simulink model "rfidet_2048ch_600mhzv2"  (R2013b) (continued)
== system rfidet_2048ch_600mhzv2 == (continued)
      | port_label('output', 1, 'q');
      | disp(['Z^-', num2str(latency)]);
  pipeline57 : Subsystem  AncestorBlock=casper_library_delays/pipeline  mask(pipeline){latency=1}
      mask help:
      | An explicitly laid-out delay line to avoid mapping to SRL16s
      | for use in pipelining to help achieve timing closure.
      mask init:
      | pipeline_init(gcb,'latency',latency);
      settings: AttributesFormatString=Latency = %<latency>
      mask Display:
      | port_label('input', 1, 'd');
      | port_label('output', 1, 'q');
      | disp(['Z^-', num2str(latency)]);
  pipeline58 : Subsystem  AncestorBlock=casper_library_delays/pipeline  mask(pipeline){latency=1}
      mask help:
      | An explicitly laid-out delay line to avoid mapping to SRL16s
      | for use in pipelining to help achieve timing closure.
      mask init:
      | pipeline_init(gcb,'latency',latency);
      settings: AttributesFormatString=Latency = %<latency>
      mask Display:
      | port_label('input', 1, 'd');
      | port_label('output', 1, 'q');
      | disp(['Z^-', num2str(latency)]);
  pipeline59 : Subsystem  AncestorBlock=casper_library_delays/pipeline  mask(pipeline){latency=1}
      mask help:
      | An explicitly laid-out delay line to avoid mapping to SRL16s
      | for use in pipelining to help achieve timing closure.
      mask init:
      | pipeline_init(gcb,'latency',latency);
      settings: AttributesFormatString=Latency = %<latency>
      mask Display:
      | port_label('input', 1, 'd');
      | port_label('output', 1, 'q');
      | disp(['Z^-', num2str(latency)]);
  pipeline6 : Subsystem  AncestorBlock=casper_library_delays/pipeline  mask(pipeline){latency=2}
      mask help:
      | An explicitly laid-out delay line to avoid mapping to SRL16s
      | for use in pipelining to help achieve timing closure.
      mask init:
      | pipeline_init(gcb,'latency',latency);
      settings: AttributesFormatString=Latency = %<latency>
      mask Display:
      | port_label('input', 1, 'd');
      | port_label('output', 1, 'q');
      | disp(['Z^-', num2str(latency)]);
  pipeline60 : Subsystem  AncestorBlock=casper_library_delays/pipeline  mask(pipeline){latency=1}
      mask help:
      | An explicitly laid-out delay line to avoid mapping to SRL16s
      | for use in pipelining to help achieve timing closure.
      mask init:
      | pipeline_init(gcb,'latency',latency);
      settings: AttributesFormatString=Latency = %<latency>
      mask Display:
      | port_label('input', 1, 'd');
      | port_label('output', 1, 'q');
      | disp(['Z^-', num2str(latency)]);
  pipeline61 : Subsystem  AncestorBlock=casper_library_delays/pipeline  mask(pipeline){latency=2}
      mask help:
      | An explicitly laid-out delay line to avoid mapping to SRL16s
      | for use in pipelining to help achieve timing closure.
      mask init:
      | pipeline_init(gcb,'latency',latency);
      settings: AttributesFormatString=Latency = %<latency>
      mask Display:
      | port_label('input', 1, 'd');
      | port_label('output', 1, 'q');
      | disp(['Z^-', num2str(latency)]);
  pipeline62 : Subsystem  AncestorBlock=casper_library_delays/pipeline  mask(pipeline){latency=2}
      mask help:
      | An explicitly laid-out delay line to avoid mapping to SRL16s
      | for use in pipelining to help achieve timing closure.
      mask init:
      | pipeline_init(gcb,'latency',latency);
      settings: AttributesFormatString=Latency = %<latency>
      mask Display:
      | port_label('input', 1, 'd');
      | port_label('output', 1, 'q');
      | disp(['Z^-', num2str(latency)]);
  pipeline63 : Subsystem  AncestorBlock=casper_library_delays/pipeline  mask(pipeline){latency=1}
      mask help:
      | An explicitly laid-out delay line to avoid mapping to SRL16s
      | for use in pipelining to help achieve timing closure.
      mask init:
      | pipeline_init(gcb,'latency',latency);
      settings: AttributesFormatString=Latency = %<latency>
      mask Display:
      | port_label('input', 1, 'd');
      | port_label('output', 1, 'q');
      | disp(['Z^-', num2str(latency)]);
  pipeline64 : Subsystem  AncestorBlock=casper_library_delays/pipeline  mask(pipeline){latency=1}
      mask help:
      | An explicitly laid-out delay line to avoid mapping to SRL16s
      | for use in pipelining to help achieve timing closure.
      mask init:
      | pipeline_init(gcb,'latency',latency);
      settings: AttributesFormatString=Latency = %<latency>
      mask Display:
      | port_label('input', 1, 'd');
      | port_label('output', 1, 'q');
      | disp(['Z^-', num2str(latency)]);
  pipeline65 : Subsystem  AncestorBlock=casper_library_delays/pipeline  mask(pipeline){latency=2}
      mask help:
      | An explicitly laid-out delay line to avoid mapping to SRL16s
      | for use in pipelining to help achieve timing closure.
      mask init:
      | pipeline_init(gcb,'latency',latency);
      settings: AttributesFormatString=Latency = %<latency>
      mask Display:
      | port_label('input', 1, 'd');
      | port_label('output', 1, 'q');
      | disp(['Z^-', num2str(latency)]);
  pipeline66 : Subsystem  AncestorBlock=casper_library_delays/pipeline  mask(pipeline){latency=2}
      mask help:
      | An explicitly laid-out delay line to avoid mapping to SRL16s
      | for use in pipelining to help achieve timing closure.
      mask init:
      | pipeline_init(gcb,'latency',latency);
      settings: AttributesFormatString=Latency = %<latency>
      mask Display:
      | port_label('input', 1, 'd');
      | port_label('output', 1, 'q');
      | disp(['Z^-', num2str(latency)]);
  pipeline67 : Subsystem  AncestorBlock=casper_library_delays/pipeline  mask(pipeline){latency=2}
      mask help:
      | An explicitly laid-out delay line to avoid mapping to SRL16s
      | for use in pipelining to help achieve timing closure.
      mask init:
      | pipeline_init(gcb,'latency',latency);
      settings: AttributesFormatString=Latency = %<latency>
      mask Display:
      | port_label('input', 1, 'd');
      | port_label('output', 1, 'q');
      | disp(['Z^-', num2str(latency)]);
  pipeline68 : Subsystem  AncestorBlock=casper_library_delays/pipeline  mask(pipeline){latency=2}
      mask help:
      | An explicitly laid-out delay line to avoid mapping to SRL16s
      | for use in pipelining to help achieve timing closure.
      mask init:
      | pipeline_init(gcb,'latency',latency);
      settings: AttributesFormatString=Latency = %<latency>
      mask Display:
      | port_label('input', 1, 'd');
      | port_label('output', 1, 'q');
      | disp(['Z^-', num2str(latency)]);
  pipeline69 : Subsystem  AncestorBlock=casper_library_delays/pipeline  mask(pipeline){latency=1}
      mask help:
      | An explicitly laid-out delay line to avoid mapping to SRL16s
      | for use in pipelining to help achieve timing closure.
      mask init:
      | pipeline_init(gcb,'latency',latency);
      settings: AttributesFormatString=Latency = %<latency>
      mask Display:
      | port_label('input', 1, 'd');
      | port_label('output', 1, 'q');
      | disp(['Z^-', num2str(latency)]);
  pipeline7 : Subsystem  AncestorBlock=casper_library_delays/pipeline  mask(pipeline){latency=1}
      mask help:
      | An explicitly laid-out delay line to avoid mapping to SRL16s
      | for use in pipelining to help achieve timing closure.
      mask init:
      | pipeline_init(gcb,'latency',latency);
      settings: AttributesFormatString=Latency = %<latency>
      mask Display:
      | port_label('input', 1, 'd');
      | port_label('output', 1, 'q');
      | disp(['Z^-', num2str(latency)]);
  pipeline70 : Subsystem  AncestorBlock=casper_library_delays/pipeline  mask(pipeline){latency=2}
      mask help:
      | An explicitly laid-out delay line to avoid mapping to SRL16s
      | for use in pipelining to help achieve timing closure.
      mask init:
      | pipeline_init(gcb,'latency',latency);
      settings: AttributesFormatString=Latency = %<latency>
      mask Display:
      | port_label('input', 1, 'd');
      | port_label('output', 1, 'q');
      | disp(['Z^-', num2str(latency)]);
  pipeline71 : Subsystem  AncestorBlock=casper_library_delays/pipeline  mask(pipeline){latency=2}
      mask help:
      | An explicitly laid-out delay line to avoid mapping to SRL16s
      | for use in pipelining to help achieve timing closure.
      mask init:
      | pipeline_init(gcb,'latency',latency);
      settings: AttributesFormatString=Latency = %<latency>
      mask Display:
      | port_label('input', 1, 'd');
      | port_label('output', 1, 'q');
      | disp(['Z^-', num2str(latency)]);
  pipeline72 : Subsystem  AncestorBlock=casper_library_delays/pipeline  mask(pipeline){latency=1}
      mask help:
      | An explicitly laid-out delay line to avoid mapping to SRL16s
      | for use in pipelining to help achieve timing closure.
      mask init:
      | pipeline_init(gcb,'latency',latency);
      settings: AttributesFormatString=Latency = %<latency>
      mask Display:
      | port_label('input', 1, 'd');
      | port_label('output', 1, 'q');
      | disp(['Z^-', num2str(latency)]);
  pipeline73 : Subsystem  AncestorBlock=casper_library_delays/pipeline  mask(pipeline){latency=1}
      mask help:
      | An explicitly laid-out delay line to avoid mapping to SRL16s
      | for use in pipelining to help achieve timing closure.
      mask init:
      | pipeline_init(gcb,'latency',latency);
      settings: AttributesFormatString=Latency = %<latency>
      mask Display:
      | port_label('input', 1, 'd');
      | port_label('output', 1, 'q');
      | disp(['Z^-', num2str(latency)]);
  pipeline77 : Subsystem  AncestorBlock=casper_library_delays/pipeline  mask(pipeline){latency=2}
      mask help:
      | An explicitly laid-out delay line to avoid mapping to SRL16s
      | for use in pipelining to help achieve timing closure.
      mask init:
      | pipeline_init(gcb,'latency',latency);
      settings: AttributesFormatString=Latency = %<latency>
      mask Display:
      | port_label('input', 1, 'd');
      | port_label('output', 1, 'q');
      | disp(['Z^-', num2str(latency)]);
  pipeline79 : Subsystem  AncestorBlock=casper_library_delays/pipeline  mask(pipeline){latency=1}
      mask help:
      | An explicitly laid-out delay line to avoid mapping to SRL16s
      | for use in pipelining to help achieve timing closure.
      mask init:
      | pipeline_init(gcb,'latency',latency);
      settings: AttributesFormatString=Latency = %<latency>
      mask Display:
      | port_label('input', 1, 'd');
      | port_label('output', 1, 'q');
      | disp(['Z^-', num2str(latency)]);
  pipeline8 : Subsystem  AncestorBlock=casper_library_delays/pipeline  mask(pipeline){latency=1}
      mask help:
      | An explicitly laid-out delay line to avoid mapping to SRL16s
      | for use in pipelining to help achieve timing closure.
      mask init:
      | pipeline_init(gcb,'latency',latency);
      settings: AttributesFormatString=Latency = %<latency>
      mask Display:
      | port_label('input', 1, 'd');
      | port_label('output', 1, 'q');
      | disp(['Z^-', num2str(latency)]);
  pipeline80 : Subsystem  AncestorBlock=casper_library_delays/pipeline  mask(pipeline){latency=1}
      mask help:
      | An explicitly laid-out delay line to avoid mapping to SRL16s
      | for use in pipelining to help achieve timing closure.
      mask init:
      | pipeline_init(gcb,'latency',latency);
      settings: AttributesFormatString=Latency = %<latency>
      mask Display:
      | port_label('input', 1, 'd');
      | port_label('output', 1, 'q');
      | disp(['Z^-', num2str(latency)]);
  pipeline81 : Subsystem  AncestorBlock=casper_library_delays/pipeline  mask(pipeline){latency=1}
      mask help:
      | An explicitly laid-out delay line to avoid mapping to SRL16s
      | for use in pipelining to help achieve timing closure.
      mask init:
      | pipeline_init(gcb,'latency',latency);
      settings: AttributesFormatString=Latency = %<latency>
      mask Display:
      | port_label('input', 1, 'd');
      | port_label('output', 1, 'q');
      | disp(['Z^-', num2str(latency)]);
  pipeline82 : Subsystem  AncestorBlock=casper_library_delays/pipeline  mask(pipeline){latency=2}
      mask help:
      | An explicitly laid-out delay line to avoid mapping to SRL16s
      | for use in pipelining to help achieve timing closure.
      mask init:
      | pipeline_init(gcb,'latency',latency);
      settings: AttributesFormatString=Latency = %<latency>
      mask Display:
      | port_label('input', 1, 'd');
      | port_label('output', 1, 'q');
      | disp(['Z^-', num2str(latency)]);
  pipeline83 : Subsystem  AncestorBlock=casper_library_delays/pipeline  mask(pipeline){latency=1}
      mask help:
      | An explicitly laid-out delay line to avoid mapping to SRL16s
      | for use in pipelining to help achieve timing closure.
      mask init:
      | pipeline_init(gcb,'latency',latency);
      settings: AttributesFormatString=Latency = %<latency>
      mask Display:
      | port_label('input', 1, 'd');
      | port_label('output', 1, 'q');
      | disp(['Z^-', num2str(latency)]);
  pipeline84 : Subsystem  AncestorBlock=casper_library_delays/pipeline  mask(pipeline){latency=1}
      mask help:
      | An explicitly laid-out delay line to avoid mapping to SRL16s
      | for use in pipelining to help achieve timing closure.
      mask init:
      | pipeline_init(gcb,'latency',latency);
      settings: AttributesFormatString=Latency = %<latency>
      mask Display:
      | port_label('input', 1, 'd');
      | port_label('output', 1, 'q');
      | disp(['Z^-', num2str(latency)]);
  pipeline85 : Subsystem  AncestorBlock=casper_library_delays/pipeline  mask(pipeline){latency=2}
      mask help:
      | An explicitly laid-out delay line to avoid mapping to SRL16s
      | for use in pipelining to help achieve timing closure.
      mask init:
      | pipeline_init(gcb,'latency',latency);
      settings: AttributesFormatString=Latency = %<latency>
      mask Display:
      | port_label('input', 1, 'd');
      | port_label('output', 1, 'q');
      | disp(['Z^-', num2str(latency)]);
  pipeline87 : Subsystem  AncestorBlock=casper_library_delays/pipeline  mask(pipeline){latency=1}
      mask help:
      | An explicitly laid-out delay line to avoid mapping to SRL16s
      | for use in pipelining to help achieve timing closure.
      mask init:
      | pipeline_init(gcb,'latency',latency);
      settings: AttributesFormatString=Latency = %<latency>
      mask Display:
      | port_label('input', 1, 'd');
      | port_label('output', 1, 'q');
      | disp(['Z^-', num2str(latency)]);
  pipeline88 : Subsystem  AncestorBlock=casper_library_delays/pipeline  mask(pipeline){latency=1}
      mask help:
      | An explicitly laid-out delay line to avoid mapping to SRL16s
      | for use in pipelining to help achieve timing closure.
      mask init:
      | pipeline_init(gcb,'latency',latency);
      settings: AttributesFormatString=Latency = %<latency>
      mask Display:
      | port_label('input', 1, 'd');
      | port_label('output', 1, 'q');
      | disp(['Z^-', num2str(latency)]);
  pipeline89 : Subsystem  AncestorBlock=casper_library_delays/pipeline  mask(pipeline){latency=1}
      mask help:
      | An explicitly laid-out delay line to avoid mapping to SRL16s
      | for use in pipelining to help achieve timing closure.
      mask init:
      | pipeline_init(gcb,'latency',latency);
      settings: AttributesFormatString=Latency = %<latency>
      mask Display:
      | port_label('input', 1, 'd');
      | port_label('output', 1, 'q');
      | disp(['Z^-', num2str(latency)]);
  pipeline9 : Subsystem  AncestorBlock=casper_library_delays/pipeline  mask(pipeline){latency=1}
      mask help:
      | An explicitly laid-out delay line to avoid mapping to SRL16s
      | for use in pipelining to help achieve timing closure.
      mask init:
      | pipeline_init(gcb,'latency',latency);
      settings: AttributesFormatString=Latency = %<latency>
      mask Display:
      | port_label('input', 1, 'd');
      | port_label('output', 1, 'q');
      | disp(['Z^-', num2str(latency)]);
  pipeline90 : Subsystem  AncestorBlock=casper_library_delays/pipeline  mask(pipeline){latency=1}
      mask help:
      | An explicitly laid-out delay line to avoid mapping to SRL16s
      | for use in pipelining to help achieve timing closure.
      mask init:
      | pipeline_init(gcb,'latency',latency);
      settings: AttributesFormatString=Latency = %<latency>
      mask Display:
      | port_label('input', 1, 'd');
      | port_label('output', 1, 'q');
      | disp(['Z^-', num2str(latency)]);
  pipeline91 : Subsystem  AncestorBlock=casper_library_delays/pipeline  mask(pipeline){latency=2}
      mask help:
      | An explicitly laid-out delay line to avoid mapping to SRL16s
      | for use in pipelining to help achieve timing closure.
      mask init:
      | pipeline_init(gcb,'latency',latency);
      settings: AttributesFormatString=Latency = %<latency>
      mask Display:
      | port_label('input', 1, 'd');
      | port_label('output', 1, 'q');
      | disp(['Z^-', num2str(latency)]);
  pipeline92 : Subsystem  AncestorBlock=casper_library_delays/pipeline  mask(pipeline){latency=1}
      mask help:
      | An explicitly laid-out delay line to avoid mapping to SRL16s
      | for use in pipelining to help achieve timing closure.
      mask init:
      | pipeline_init(gcb,'latency',latency);
      settings: AttributesFormatString=Latency = %<latency>
      mask Display:
      | port_label('input', 1, 'd');
      | port_label('output', 1, 'q');
      | disp(['Z^-', num2str(latency)]);
  pipeline93 : Subsystem  AncestorBlock=casper_library_delays/pipeline  mask(pipeline){latency=1}
      mask help:
      | An explicitly laid-out delay line to avoid mapping to SRL16s
      | for use in pipelining to help achieve timing closure.
      mask init:
      | pipeline_init(gcb,'latency',latency);
      settings: AttributesFormatString=Latency = %<latency>
      mask Display:
      | port_label('input', 1, 'd');
      | port_label('output', 1, 'q');
      | disp(['Z^-', num2str(latency)]);
  pipeline94 : Subsystem  AncestorBlock=casper_library_delays/pipeline  mask(pipeline){latency=2}
      mask help:
      | An explicitly laid-out delay line to avoid mapping to SRL16s
      | for use in pipelining to help achieve timing closure.
      mask init:
      | pipeline_init(gcb,'latency',latency);
      settings: AttributesFormatString=Latency = %<latency>
      mask Display:
      | port_label('input', 1, 'd');
      | port_label('output', 1, 'q');
      | disp(['Z^-', num2str(latency)]);
  pipeline96 : Subsystem  AncestorBlock=casper_library_delays/pipeline  mask(pipeline){latency=1}
      mask help:
      | An explicitly laid-out delay line to avoid mapping to SRL16s
      | for use in pipelining to help achieve timing closure.
      mask init:
      | pipeline_init(gcb,'latency',latency);
      settings: AttributesFormatString=Latency = %<latency>
      mask Display:
      | port_label('input', 1, 'd');
      | port_label('output', 1, 'q');
      | disp(['Z^-', num2str(latency)]);
  pipeline97 : Subsystem  AncestorBlock=casper_library_delays/pipeline  mask(pipeline){latency=1}
      mask help:
      | An explicitly laid-out delay line to avoid mapping to SRL16s
      | for use in pipelining to help achieve timing closure.
      mask init:
      | pipeline_init(gcb,'latency',latency);
      settings: AttributesFormatString=Latency = %<latency>
      mask Display:
      | port_label('input', 1, 'd');
      | port_label('output', 1, 'q');
      | disp(['Z^-', num2str(latency)]);
  pipeline98 : Subsystem  AncestorBlock=casper_library_delays/pipeline  mask(pipeline){latency=2}
      mask help:
      | An explicitly laid-out delay line to avoid mapping to SRL16s
      | for use in pipelining to help achieve timing closure.
      mask init:
      | pipeline_init(gcb,'latency',latency);
      settings: AttributesFormatString=Latency = %<latency>
      mask Display:
      | port_label('input', 1, 'd');
      | port_label('output', 1, 'q');
      | disp(['Z^-', num2str(latency)]);
  pipeline99 : Subsystem  AncestorBlock=casper_library_delays/pipeline  mask(pipeline){latency=1}
      mask help:
      | An explicitly laid-out delay line to avoid mapping to SRL16s
      | for use in pipelining to help achieve timing closure.
      mask init:
      | pipeline_init(gcb,'latency',latency);
      settings: AttributesFormatString=Latency = %<latency>
      mask Display:
      | port_label('input', 1, 'd');
      | port_label('output', 1, 'q');
      | disp(['Z^-', num2str(latency)]);
  power{1..7,12} (8 alike) : casper_library_misc/power  BitWidth=18  add_latency=2  mult_latency=3
  signed_shifter{,1..15} (16 alike) : xbsIndex_r4/Black Box  init_code=shifter_signed_config  sim_method=Inactive  verbose=off  block_type=blackbox2
  simple_bram_vacc0_0 : Subsystem  AncestorBlock=casper_library_accumulators/simple_bram_vacc  mask(simple_bram_vacc){vec_len=512, arith_type=Unsigned, n_bits=64, bin_pt=35}
      mask prompts: vec_len "Vector length:"; arith_type "Output type:"; n_bits "Bit width (output):"; bin_pt "Binary point (output):"
      mask help: This simple vector accumulator is based on a shift register. It outputs its previous accumulated vector after a new_acc pulse is received.
      mask init:
      | simple_bram_vacc_init(gcb, ...
      |     'vec_len', vec_len, ...
      |     'arith_type', arith_type, ...
      |     'n_bits', n_bits, ...
      |     'bin_pt', bin_pt);
      mask TypeOptions arith_type: Signed | Unsigned
  simple_bram_vacc0_1 : Subsystem  AncestorBlock=casper_library_accumulators/simple_bram_vacc  mask(simple_bram_vacc){vec_len=512, arith_type=Unsigned, n_bits=64, bin_pt=35}
      mask prompts: vec_len "Vector length:"; arith_type "Output type:"; n_bits "Bit width (output):"; bin_pt "Binary point (output):"
      mask help: This simple vector accumulator is based on a shift register. It outputs its previous accumulated vector after a new_acc pulse is received.
      mask init:
      | simple_bram_vacc_init(gcb, ...
      |     'vec_len', vec_len, ...
      |     'arith_type', arith_type, ...
      |     'n_bits', n_bits, ...
      |     'bin_pt', bin_pt);
      mask TypeOptions arith_type: Signed | Unsigned
  simple_bram_vacc0_2 : Subsystem  AncestorBlock=casper_library_accumulators/simple_bram_vacc  mask(simple_bram_vacc){vec_len=512, arith_type=Unsigned, n_bits=64, bin_pt=35}
      mask prompts: vec_len "Vector length:"; arith_type "Output type:"; n_bits "Bit width (output):"; bin_pt "Binary point (output):"
      mask help: This simple vector accumulator is based on a shift register. It outputs its previous accumulated vector after a new_acc pulse is received.
      mask init:
      | simple_bram_vacc_init(gcb, ...
      |     'vec_len', vec_len, ...
      |     'arith_type', arith_type, ...
      |     'n_bits', n_bits, ...
      |     'bin_pt', bin_pt);
      mask TypeOptions arith_type: Signed | Unsigned
  simple_bram_vacc0_3 : Subsystem  AncestorBlock=casper_library_accumulators/simple_bram_vacc  mask(simple_bram_vacc){vec_len=512, arith_type=Unsigned, n_bits=64, bin_pt=35}
      mask prompts: vec_len "Vector length:"; arith_type "Output type:"; n_bits "Bit width (output):"; bin_pt "Binary point (output):"
      mask help: This simple vector accumulator is based on a shift register. It outputs its previous accumulated vector after a new_acc pulse is received.
      mask init:
      | simple_bram_vacc_init(gcb, ...
      |     'vec_len', vec_len, ...
      |     'arith_type', arith_type, ...
      |     'n_bits', n_bits, ...
      |     'bin_pt', bin_pt);
      mask TypeOptions arith_type: Signed | Unsigned
  simple_bram_vacc0_4 : Subsystem  AncestorBlock=casper_library_accumulators/simple_bram_vacc  mask(simple_bram_vacc){vec_len=512, arith_type=Unsigned, n_bits=64, bin_pt=35}
      mask prompts: vec_len "Vector length:"; arith_type "Output type:"; n_bits "Bit width (output):"; bin_pt "Binary point (output):"
      mask help: This simple vector accumulator is based on a shift register. It outputs its previous accumulated vector after a new_acc pulse is received.
      mask init:
      | simple_bram_vacc_init(gcb, ...
      |     'vec_len', vec_len, ...
      |     'arith_type', arith_type, ...
      |     'n_bits', n_bits, ...
      |     'bin_pt', bin_pt);
      mask TypeOptions arith_type: Signed | Unsigned
  simple_bram_vacc0_5 : Subsystem  AncestorBlock=casper_library_accumulators/simple_bram_vacc  mask(simple_bram_vacc){vec_len=512, arith_type=Unsigned, n_bits=64, bin_pt=35}
      mask prompts: vec_len "Vector length:"; arith_type "Output type:"; n_bits "Bit width (output):"; bin_pt "Binary point (output):"
      mask help: This simple vector accumulator is based on a shift register. It outputs its previous accumulated vector after a new_acc pulse is received.
      mask init:
      | simple_bram_vacc_init(gcb, ...
      |     'vec_len', vec_len, ...
      |     'arith_type', arith_type, ...
      |     'n_bits', n_bits, ...
      |     'bin_pt', bin_pt);
      mask TypeOptions arith_type: Signed | Unsigned
  simple_bram_vacc0_6 : Subsystem  AncestorBlock=casper_library_accumulators/simple_bram_vacc  mask(simple_bram_vacc){vec_len=512, arith_type=Unsigned, n_bits=64, bin_pt=35}
      mask prompts: vec_len "Vector length:"; arith_type "Output type:"; n_bits "Bit width (output):"; bin_pt "Binary point (output):"
      mask help: This simple vector accumulator is based on a shift register. It outputs its previous accumulated vector after a new_acc pulse is received.
      mask init:
      | simple_bram_vacc_init(gcb, ...
      |     'vec_len', vec_len, ...
      |     'arith_type', arith_type, ...
      |     'n_bits', n_bits, ...
      |     'bin_pt', bin_pt);
      mask TypeOptions arith_type: Signed | Unsigned
  simple_bram_vacc0_7 : Subsystem  AncestorBlock=casper_library_accumulators/simple_bram_vacc  mask(simple_bram_vacc){vec_len=512, arith_type=Unsigned, n_bits=64, bin_pt=35}
      mask prompts: vec_len "Vector length:"; arith_type "Output type:"; n_bits "Bit width (output):"; bin_pt "Binary point (output):"
      mask help: This simple vector accumulator is based on a shift register. It outputs its previous accumulated vector after a new_acc pulse is received.
      mask init:
      | simple_bram_vacc_init(gcb, ...
      |     'vec_len', vec_len, ...
      |     'arith_type', arith_type, ...
      |     'n_bits', n_bits, ...
      |     'bin_pt', bin_pt);
      mask TypeOptions arith_type: Signed | Unsigned
  sync_gen : Subsystem  AncestorBlock=casper_library_misc/sync_gen  mask(sync_gen){sim_acc_len=1, fft_size=2^12, fft_simult_inputs=8, pfb_fir_taps=4, reorder_vec=[2 2 9], scale=1}
      mask prompts: sim_acc_len "Simulation Accumulation Length"; fft_size "FFT Size"; fft_simult_inputs "Simultaneous Inputs (FFT)"; pfb_fir_taps "Taps in pfb_fir (use 1 if no pfb_fir)"; reorder_vec "Reorder Orders (e.g. [2 2 2])"
      mask help:
      | This block computes the sync period based on system parameters as
      | described in Casper memo.  Detailed descritions of the block's
      | variables can be found in the 'Block Documentation' section of
      | the CASPER wiki.
      mask init:
      | sync_gen_init(gcb, ...
      |     'sim_acc_len', sim_acc_len, ...
      |     'fft_size', fft_size, ...
      |     'fft_simult_inputs', fft_simult_inputs,...
      |     'pfb_fir_taps', pfb_fir_taps, ...
      |     'reorder_vec', reorder_vec, ...
      |     'scale', scale);
      settings: AttributesFormatString=sim_sync_period=36864
      mask Help:
      | SyncPeriod=LCM(reorder_vec)*(acc_num)*(pfb_fir_len)*(fft_size/fft_simult_inputs)
      | 
      | See CASPER sync pulse memo for more details.
  trigger : Subsystem  Tag=xps:sw_reg  AncestorBlock=xps_library/software_register  mask(swreg){io_dir=From Processor, io_delay=0, sample_period=1, names=reg, bitwidths=32, bin_pts=0, arith_types=0, sim_port=off, show_format=on}
      mask prompts: io_dir "I/O direction"; io_delay "I/O delay"; sample_period "Sample period"; names "Bitfield names [msb...lsb]"; bitwidths "Bitfield widths"; bin_pts "Bitfield binary pts"; arith_types "Bitfield types, ufix=0, fix=1, bool=2"; sim_port "Provide sim input/output?"; show_format "Print format string?"
      mask help: A 32-bit software-accessible register. Can be divided into bitfields of varying widths and types using the fields in the block mask.
      mask init:
      | try
      |     swreg_init(gcb)
      | catch ex
      |     disp(sprintf('%s: %s', gcb, ex.message))
      |     errordlg(ex.message)
      | end
      settings: AttributesFormatString=1input:uf32.0 = 32 bits
      mask TypeOptions io_dir: To Processor | From Processor
      mask Callback io_dir:
      | mask_enables = get_param(gcb, 'MaskEnables');
      | mask_names = get_param(gcb, 'MaskNames');
      | if strcmp(get_param(gcb, 'io_dir'), 'To Processor'),
      |     mask_enables{ismember(mask_names, 'sample_period')} = 'off';
      | else
      |     mask_enables{ismember(mask_names, 'sample_period')} = 'on';
      | end
      | set_param(gcb, 'MaskEnables', mask_enables);
  wires:
  cnt_rst/1(in_reg) -> pipeline5/1(d)
  pipeline5/1(q) -> Slice/1
  Slice/1 -> [cnt_rst]
  acc_len/1(in_reg) -> pipeline6/1(d)
  pipeline6/1(q) -> [acc_len]
  fft_wideband_real1/6(of) -> Terminator4/1
  Constant2/1 -> fft_wideband_real1/2(shift)
  Delay15/1 -> fft_wideband_real1/1(sync)
  Shift/1 -> fft_wideband_real1/3(in00)
  Shift1/1 -> fft_wideband_real1/4(in01)
  Shift2/1 -> fft_wideband_real1/5(in02)
  Shift3/1 -> fft_wideband_real1/6(in03)
  Shift4/1 -> fft_wideband_real1/7(in04)
  Shift5/1 -> fft_wideband_real1/8(in05)
  Shift6/1 -> fft_wideband_real1/9(in06)
  Shift7/1 -> fft_wideband_real1/10(in07)
  Delay16/1 -> pipeline20/1(d)
  Band-Limited White Noise2/1 -> asiaa_adc5g0/1(sim_a)
  bus_create/1(bus_out) -> pipeline21/1(d)
  Constant5/1 -> adcsnap0/2(we), adcsnap0/3(trig)
  pipeline21/1(q) -> adcsnap0/1(din)
  detector_gain/1(in_reg) -> pipeline47/1(d)
  Constant13/1 -> asiaa_adc5g0/3(sim_sync)
  dout0_0/1(data_out) -> Terminator1/1
  dout0_1/1(data_out) -> Terminator2/1
  dout0_2/1(data_out) -> Terminator3/1
  dout0_3/1(data_out) -> Terminator9/1
  Reinterpret1/1 -> Delay1/1
  Reinterpret2/1 -> Delay3/1
  Reinterpret3/1 -> Delay5/1
  Reinterpret12/1 -> Delay11/1
  simple_bram_vacc0_0/1(dout) -> pipeline150/1(d)
  simple_bram_vacc0_0/2(valid) -> pipeline151/1(d)
  simple_bram_vacc0_1/2(valid) -> pipeline154/1(d)
  simple_bram_vacc0_1/1(dout) -> pipeline153/1(d)
  simple_bram_vacc0_2/2(valid) -> pipeline156/1(d)
  simple_bram_vacc0_2/1(dout) -> pipeline155/1(d)
  simple_bram_vacc0_3/1(dout) -> pipeline157/1(d)
  simple_bram_vacc0_3/2(valid) -> pipeline158/1(d)
  acc_cntrl0/1(new_acc) -> pipeline88/1(d), pipeline9/1(d), pipeline7/1(d), pipeline142/1(d)
  pipeline2/1(q) -> simple_bram_vacc0_0/2(din)
  pipeline8/1(q) -> simple_bram_vacc0_1/2(din)
  pipeline80/1(q) -> simple_bram_vacc0_2/2(din)
  pipeline87/1(q) -> simple_bram_vacc0_3/2(din)
  [acc_len] -> acc_cntrl0/1(acc_len)
  [cnt_rst] -> acc_cntrl0/3(rst)
  Delay6/1 -> pipeline2/1(d)
  Delay7/1 -> pipeline8/1(d)
  Delay8/1 -> pipeline80/1(d)
  Delay9/1 -> pipeline87/1(d)
  pipeline3/1(q) -> Delay6/1
  pipeline64/1(q) -> Delay7/1
  pipeline11/1(q) -> Delay8/1
  pipeline10/1(q) -> Delay9/1
  power1/1 -> pipeline3/1(d)
  power2/1 -> pipeline64/1(d)
  power3/1 -> pipeline11/1(d)
  power12/1 -> pipeline10/1(d)
  pipeline4/1(q) -> acc_cntrl0/2(sync)
  Delay36/1 -> pipeline4/1(d)
  pipeline47/1(q) -> Slice10/1
  conv_bus0/1(Out1) -> internal_oor/1(In1), bus_create/1(in1), pipeline19/1(d)
  conv_bus0/6(Out6) -> internal_oor/6(In6), bus_create/6(in6), pipeline14/1(d)
  conv_bus0/7(Out7) -> internal_oor/7(In7), bus_create/7(in7), pipeline13/1(d)
  conv_bus0/8(Out8) -> internal_oor/8(In8), bus_create/8(in8), pipeline12/1(d)
  conv_bus0/4(Out4) -> internal_oor/4(In4), bus_create/4(in4), pipeline16/1(d)
  conv_bus0/2(Out2) -> internal_oor/2(In2), bus_create/2(in2), pipeline18/1(d)
  conv_bus0/3(Out3) -> internal_oor/3(In3), bus_create/3(in3), pipeline17/1(d)
  conv_bus0/5(Out5) -> internal_oor/5(In5), bus_create/5(in5), pipeline15/1(d)
  asiaa_adc5g0/1(a0) -> conv_bus0/1(In1)
  asiaa_adc5g0/2(a1) -> conv_bus0/2(In2)
  asiaa_adc5g0/3(a2) -> conv_bus0/3(In3)
  asiaa_adc5g0/4(a3) -> conv_bus0/4(In4)
  asiaa_adc5g0/5(a4) -> conv_bus0/5(In5)
  asiaa_adc5g0/6(a5) -> conv_bus0/6(In6)
  asiaa_adc5g0/7(a6) -> conv_bus0/7(In7)
  asiaa_adc5g0/8(a7) -> conv_bus0/8(In8)
  pipeline20/1(q) -> pfb_fir_real0/1(sync)
  pipeline19/1(q) -> pfb_fir_real0/2(pol1_in1)
  pipeline18/1(q) -> pfb_fir_real0/3(pol1_in2)
  pipeline17/1(q) -> pfb_fir_real0/4(pol1_in3)
  pipeline16/1(q) -> pfb_fir_real0/5(pol1_in4)
  pipeline15/1(q) -> pfb_fir_real0/6(pol1_in5)
  pipeline14/1(q) -> pfb_fir_real0/7(pol1_in6)
  pipeline13/1(q) -> pfb_fir_real0/8(pol1_in7)
  pipeline12/1(q) -> pfb_fir_real0/9(pol1_in8)
  pfb_fir_real0/2(pol1_out1) -> Shift/1
  pfb_fir_real0/3(pol1_out2) -> Shift1/1
  pfb_fir_real0/4(pol1_out3) -> Shift2/1
  pfb_fir_real0/5(pol1_out4) -> Shift3/1
  pfb_fir_real0/6(pol1_out5) -> Shift4/1
  pfb_fir_real0/7(pol1_out6) -> Shift5/1
  pfb_fir_real0/8(pol1_out7) -> Shift6/1
  pfb_fir_real0/9(pol1_out8) -> Shift7/1
  pfb_fir_real0/1(sync_out) -> Delay15/1
  [sync_out] -> Delay16/1
  sync_gen/1(sync_out) -> [sync_out]
  [cnt_rst] -> internal_oor/9(rst)
  internal_oor/1(out_of_range) -> led_4/1
  led_4/1 -> Terminator29/1
  pipeline88/1(q) -> simple_bram_vacc0_3/1(new_acc)
  pipeline9/1(q) -> simple_bram_vacc0_2/1(new_acc)
  pipeline7/1(q) -> simple_bram_vacc0_1/1(new_acc)
  pipeline142/1(q) -> simple_bram_vacc0_0/1(new_acc)
  pipeline65/1(q) -> power12/1
  pipeline66/1(q) -> power3/1
  pipeline67/1(q) -> power2/1
  pipeline68/1(q) -> power1/1
  trigger/1(in_reg) -> pipeline242/1(d)
  pipeline242/1(q) -> Slice1/1
  Slice1/1 -> [trigger]
  [detector_gain] -> signed_shifter/2
  Delay3/1 -> Delay52/1
  Delay11/1 -> Delay50/1
  Delay5/1 -> Delay51/1
  Delay1/1 -> Delay54/1
  doutsl0_0/1(data_out) -> Terminator6/1
  doutsl0_1/1(data_out) -> Terminator14/1
  doutsl0_2/1(data_out) -> Terminator8/1
  doutsl0_3/1(data_out) -> Terminator7/1
  Slice5/1 -> Delay53/1, Mult3/1
  Slice4/1 -> Delay47/1, Mult2/1
  Slice3/1 -> Delay49/1, Mult1/1
  Slice2/1 -> Delay48/1, Mult/1
  Convert/1 -> doutsl0_0/2(data_in)
  Convert1/1 -> doutsl0_1/2(data_in)
  Convert3/1 -> doutsl0_3/2(data_in)
  Convert2/1 -> doutsl0_2/2(data_in)
  Slice10/1 -> [detector_gain]
  signed_shifter/1 -> Slice2/1
  signed_shifter1/1 -> Slice3/1
  signed_shifter2/1 -> Slice4/1
  signed_shifter3/1 -> Slice5/1
  [detector_gain] -> signed_shifter1/2
  [detector_gain] -> signed_shifter3/2
  [detector_gain] -> signed_shifter2/2
  fft_wideband_real1/3(out01) -> cmult2/1(a), pipeline67/1(d)
  fft_wideband_real1/4(out02) -> cmult3/1(a), pipeline66/1(d)
  fft_wideband_real1/5(out03) -> cmult4/1(a), pipeline65/1(d)
  Band-Limited White Noise1/1 -> asiaa_adc5g0/2(sim_c)
  pipeline151/1(q) -> Delay2/1
  Delay2/1 -> Delay56/1
  freeze_cntr2/1 -> dout0_1/1(addr), dout0_2/1(addr), dout0_3/1(addr), doutsl0_0/1(addr), doutsl0_1/1(addr), doutsl0_3/1(addr), doutsl0_2/1(addr), dout0_0/1(addr)
  fft_wideband_real2/6(of) -> Terminator17/1
  Constant4/1 -> fft_wideband_real2/2(shift)
  Delay19/1 -> fft_wideband_real2/1(sync)
  Shift8/1 -> fft_wideband_real2/3(in00)
  Shift9/1 -> fft_wideband_real2/4(in01)
  Shift10/1 -> fft_wideband_real2/5(in02)
  Shift11/1 -> fft_wideband_real2/6(in03)
  Shift12/1 -> fft_wideband_real2/7(in04)
  Shift13/1 -> fft_wideband_real2/8(in05)
  Shift14/1 -> fft_wideband_real2/9(in06)
  Shift15/1 -> fft_wideband_real2/10(in07)
  Delay20/1 -> pipeline44/1(d)
  bus_create1/1(bus_out) -> pipeline45/1(d)
  Constant6/1 -> adcsnap1/3(trig), adcsnap1/2(we)
  pipeline45/1(q) -> adcsnap1/1(din)
  Reinterpret4/1 -> Delay13/1
  Reinterpret6/1 -> Delay24/1
  Reinterpret7/1 -> Delay27/1
  Reinterpret5/1 -> Delay17/1
  simple_bram_vacc0_4/1(dout) -> pipeline30/1(d)
  simple_bram_vacc0_4/2(valid) -> pipeline31/1(d)
  simple_bram_vacc0_5/2(valid) -> pipeline34/1(d)
  simple_bram_vacc0_5/1(dout) -> pipeline33/1(d)
  simple_bram_vacc0_6/2(valid) -> pipeline36/1(d)
  simple_bram_vacc0_6/1(dout) -> pipeline35/1(d)
  simple_bram_vacc0_7/1(dout) -> pipeline37/1(d)
  simple_bram_vacc0_7/2(valid) -> pipeline38/1(d)
  acc_cntrl1/1(new_acc) -> pipeline28/1(d), pipeline54/1(d), pipeline60/1(d), pipeline59/1(d)
  pipeline43/1(q) -> simple_bram_vacc0_4/2(din)
  pipeline56/1(q) -> simple_bram_vacc0_5/2(din)
  pipeline57/1(q) -> simple_bram_vacc0_6/2(din)
  pipeline58/1(q) -> simple_bram_vacc0_7/2(din)
  [acc_len] -> acc_cntrl1/1(acc_len)
  [cnt_rst] -> acc_cntrl1/3(rst)
  Delay28/1 -> pipeline43/1(d)
  Delay29/1 -> pipeline56/1(d)
  Delay30/1 -> pipeline57/1(d)
  Delay31/1 -> pipeline58/1(d)
  pipeline46/1(q) -> Delay28/1
  pipeline49/1(q) -> Delay29/1
  pipeline24/1(q) -> Delay30/1
  pipeline23/1(q) -> Delay31/1
  power4/1 -> pipeline46/1(d)
  power6/1 -> pipeline49/1(d)
  power7/1 -> pipeline24/1(d)
  power5/1 -> pipeline23/1(d)
  pipeline48/1(q) -> acc_cntrl1/2(sync)
  Delay25/1 -> pipeline48/1(d)
  conv_bus1/1(Out1) -> pipeline42/1(d), bus_create1/1(in1), internal_oor1/1(In1)
  conv_bus1/6(Out6) -> pipeline27/1(d), bus_create1/6(in6), internal_oor1/6(In6)
  conv_bus1/7(Out7) -> pipeline26/1(d), bus_create1/7(in7), internal_oor1/7(In7)
  conv_bus1/8(Out8) -> pipeline25/1(d), bus_create1/8(in8), internal_oor1/8(In8)
  conv_bus1/4(Out4) -> pipeline39/1(d), bus_create1/4(in4), internal_oor1/4(In4)
  conv_bus1/2(Out2) -> pipeline41/1(d), bus_create1/2(in2), internal_oor1/2(In2)
  conv_bus1/3(Out3) -> pipeline40/1(d), bus_create1/3(in3), internal_oor1/3(In3)
  conv_bus1/5(Out5) -> pipeline29/1(d), bus_create1/5(in5), internal_oor1/5(In5)
  pipeline44/1(q) -> pfb_fir_real1/1(sync)
  pipeline42/1(q) -> pfb_fir_real1/2(pol1_in1)
  pipeline41/1(q) -> pfb_fir_real1/3(pol1_in2)
  pipeline40/1(q) -> pfb_fir_real1/4(pol1_in3)
  pipeline39/1(q) -> pfb_fir_real1/5(pol1_in4)
  pipeline29/1(q) -> pfb_fir_real1/6(pol1_in5)
  pipeline27/1(q) -> pfb_fir_real1/7(pol1_in6)
  pipeline26/1(q) -> pfb_fir_real1/8(pol1_in7)
  pipeline25/1(q) -> pfb_fir_real1/9(pol1_in8)
  pfb_fir_real1/2(pol1_out1) -> Shift8/1
  pfb_fir_real1/3(pol1_out2) -> Shift9/1
  pfb_fir_real1/4(pol1_out3) -> Shift10/1
  pfb_fir_real1/5(pol1_out4) -> Shift11/1
  pfb_fir_real1/6(pol1_out5) -> Shift12/1
  pfb_fir_real1/7(pol1_out6) -> Shift13/1
  pfb_fir_real1/8(pol1_out7) -> Shift14/1
  pfb_fir_real1/9(pol1_out8) -> Shift15/1
  pfb_fir_real1/1(sync_out) -> Delay19/1
  [sync_out] -> Delay20/1
  [cnt_rst] -> internal_oor1/9(rst)
  internal_oor1/1(out_of_range) -> led_5/1
  led_5/1 -> Terminator15/1
  pipeline59/1(q) -> simple_bram_vacc0_7/1(new_acc)
  pipeline60/1(q) -> simple_bram_vacc0_6/1(new_acc)
  pipeline54/1(q) -> simple_bram_vacc0_5/1(new_acc)
  pipeline28/1(q) -> simple_bram_vacc0_4/1(new_acc)
  pipeline53/1(q) -> power5/1
  pipeline50/1(q) -> power7/1
  pipeline51/1(q) -> power6/1
  pipeline52/1(q) -> power4/1
  pipeline55/1(q) -> Delay25/1
  [detector_gain] -> signed_shifter4/2
  Slice9/1 -> Delay4/1, Mult3/2
  Slice8/1 -> Delay10/1, Mult2/2
  Slice7/1 -> Delay21/1, Mult1/2
  Slice6/1 -> Delay12/1, Mult/2
  signed_shifter4/1 -> Slice6/1
  signed_shifter5/1 -> Slice7/1
  signed_shifter6/1 -> Slice8/1
  signed_shifter7/1 -> Slice9/1
  [detector_gain] -> signed_shifter5/2
  [detector_gain] -> signed_shifter7/2
  [detector_gain] -> signed_shifter6/2
  fft_wideband_real2/1(sync_out) -> pipeline55/1(d)
  fft_wideband_real2/2(out00) -> pipeline52/1(d), cmult1/2(b)
  fft_wideband_real2/3(out01) -> pipeline51/1(d), cmult2/2(b)
  fft_wideband_real2/4(out02) -> pipeline50/1(d), cmult3/2(b)
  fft_wideband_real2/5(out03) -> cmult4/2(b), pipeline53/1(d)
  pipeline31/1(q) -> Delay22/1
  asiaa_adc5g0/9(c0) -> conv_bus1/1(In1)
  asiaa_adc5g0/10(c1) -> conv_bus1/2(In2)
  asiaa_adc5g0/11(c2) -> conv_bus1/3(In3)
  asiaa_adc5g0/12(c3) -> conv_bus1/4(In4)
  asiaa_adc5g0/13(c4) -> conv_bus1/5(In5)
  asiaa_adc5g0/14(c5) -> conv_bus1/6(In6)
  asiaa_adc5g0/15(c6) -> conv_bus1/7(In7)
  asiaa_adc5g0/16(c7) -> conv_bus1/8(In8)
  asiaa_adc5g0/17(sync_out) -> Terminator5/1
  pipeline70/1(q) -> Delay36/1
  bram_corr_re_0/1(dout) -> pipeline61/1(d)
  pipeline63/1(q) -> bram_corr_re_0/2(din)
  [acc_len] -> acc_cntrl2/1(acc_len)
  [cnt_rst] -> acc_cntrl2/3(rst)
  Delay34/1 -> pipeline63/1(d)
  pipeline69/1(q) -> bram_corr_re_0/1(new_acc)
  c_to_ri/1 -> Delay34/1
  bram_corr_im_0/1(dout) -> pipeline71/1(d)
  bram_corr_im_0/2(valid) -> Terminator31/1
  pipeline72/1(q) -> bram_corr_im_0/2(din)
  pipeline73/1(q) -> bram_corr_im_0/1(new_acc)
  c_to_ri/2 -> Delay33/1
  Delay33/1 -> pipeline72/1(d)
  bram_corr_re_0/2(valid) -> pipeline62/1(d)
  cmult1/1(ab) -> c_to_ri/1
  fft_wideband_real1/2(out00) -> pipeline68/1(d), cmult1/1(a)
  fft_wideband_real1/1(sync_out) -> pipeline70/1(d), acc_cntrl2/2(sync)
  [detector_gain] -> signed_shifter8/2
  cmult2/1(ab) -> c_to_ri1/1
  cmult3/1(ab) -> c_to_ri2/1
  cmult4/1(ab) -> c_to_ri3/1
  bram_corr_re_1/1(dout) -> pipeline77/1(d)
  pipeline79/1(q) -> bram_corr_re_1/2(din)
  Delay39/1 -> pipeline79/1(d)
  pipeline81/1(q) -> bram_corr_re_1/1(new_acc)
  bram_corr_im_1/1(dout) -> pipeline82/1(d)
  bram_corr_im_1/2(valid) -> Terminator20/1
  pipeline83/1(q) -> bram_corr_im_1/2(din)
  pipeline84/1(q) -> bram_corr_im_1/1(new_acc)
  Delay35/1 -> pipeline83/1(d)
  c_to_ri1/1 -> Delay39/1
  c_to_ri1/2 -> Delay35/1
  bram_corr_re_2/1(dout) -> pipeline85/1(d)
  pipeline89/1(q) -> bram_corr_re_2/2(din)
  Delay41/1 -> pipeline89/1(d)
  pipeline90/1(q) -> bram_corr_re_2/1(new_acc)
  c_to_ri2/1 -> Delay41/1
  bram_corr_im_2/1(dout) -> pipeline91/1(d)
  bram_corr_im_2/2(valid) -> Terminator22/1
  pipeline92/1(q) -> bram_corr_im_2/2(din)
  pipeline93/1(q) -> bram_corr_im_2/1(new_acc)
  c_to_ri2/2 -> Delay40/1
  Delay40/1 -> pipeline92/1(d)
  bram_corr_re_3/1(dout) -> pipeline94/1(d)
  pipeline96/1(q) -> bram_corr_re_3/2(din)
  Delay43/1 -> pipeline96/1(d)
  pipeline97/1(q) -> bram_corr_re_3/1(new_acc)
  acc_cntrl2/1(new_acc) -> pipeline69/1(d), pipeline97/1(d), pipeline100/1(d), pipeline93/1(d), pipeline90/1(d), pipeline84/1(d), pipeline81/1(d), pipeline73/1(d)
  bram_corr_im_3/1(dout) -> pipeline98/1(d)
  bram_corr_im_3/2(valid) -> Terminator21/1
  pipeline99/1(q) -> bram_corr_im_3/2(din)
  pipeline100/1(q) -> bram_corr_im_3/1(new_acc)
  Delay42/1 -> pipeline99/1(d)
  c_to_ri3/1 -> Delay43/1
  c_to_ri3/2 -> Delay42/1
  bram_corr_re_3/2(valid) -> Terminator23/1
  bram_corr_re_2/2(valid) -> Terminator24/1
  bram_corr_re_1/2(valid) -> Terminator25/1
  pipeline30/1(q) -> signed_shifter4/1, Reinterpret4/1
  pipeline33/1(q) -> signed_shifter5/1, Reinterpret6/1
  pipeline37/1(q) -> signed_shifter7/1, Reinterpret5/1
  pipeline35/1(q) -> signed_shifter6/1, Reinterpret7/1
  pipeline153/1(q) -> signed_shifter1/1, Reinterpret2/1
  pipeline157/1(q) -> signed_shifter3/1, Reinterpret12/1
  pipeline155/1(q) -> signed_shifter2/1, Reinterpret3/1
  pipeline150/1(q) -> signed_shifter/1, Reinterpret1/1
  pipeline61/1(q) -> signed_shifter8/1
  pipeline62/1(q) -> Delay44/1
  Delay44/1 -> Delay45/1
  [detector_gain] -> signed_shifter9/2
  [detector_gain] -> signed_shifter10/2
  [detector_gain] -> signed_shifter11/2
  [detector_gain] -> signed_shifter14/2
  [detector_gain] -> signed_shifter15/2
  [detector_gain] -> signed_shifter12/2
  [detector_gain] -> signed_shifter13/2
  pipeline98/1(q) -> signed_shifter13/1
  pipeline94/1(q) -> signed_shifter12/1
  pipeline85/1(q) -> signed_shifter14/1
  pipeline82/1(q) -> signed_shifter11/1
  pipeline91/1(q) -> signed_shifter15/1
  pipeline77/1(q) -> signed_shifter10/1
  pipeline71/1(q) -> signed_shifter9/1
  freeze_cntr2/3 -> Terminator40/1
  [trigger] -> freeze_cntr2/2
  freeze_cntr2/2 -> dout0_2/3(we), dout0_3/3(we), doutsl0_0/3(we), doutsl0_1/3(we), doutsl0_2/3(we), doutsl0_3/3(we), dout0_1/3(we), dout0_0/3(we)
  dout1_0/1(data_out) -> Terminator11/1
  dout1_1/1(data_out) -> Terminator13/1
  dout1_2/1(data_out) -> Terminator16/1
  dout1_3/1(data_out) -> Terminator38/1
  Delay24/1 -> Delay38/1
  Delay17/1 -> Delay32/1
  Delay27/1 -> Delay37/1
  Delay13/1 -> Delay46/1
  doutsl1_0/1(data_out) -> Terminator18/1
  doutsl1_1/1(data_out) -> Terminator12/1
  doutsl1_2/1(data_out) -> Terminator27/1
  doutsl1_3/1(data_out) -> Terminator19/1
  Delay22/1 -> Delay55/1, Delay57/1
  freeze_cntr1/1 -> dout1_0/1(addr), dout1_1/1(addr), dout1_2/1(addr), dout1_3/1(addr), doutsl1_1/1(addr), doutsl1_3/1(addr), doutsl1_2/1(addr), doutsl1_0/1(addr)
  freeze_cntr1/3 -> Terminator28/1
  [trigger] -> freeze_cntr1/2
  freeze_cntr1/2 -> dout1_0/3(we), dout1_1/3(we), dout1_2/3(we), dout1_3/3(we), doutsl1_1/3(we), doutsl1_2/3(we), doutsl1_3/3(we), doutsl1_0/3(we)
  Convert4/1 -> doutsl1_0/2(data_in)
  Convert5/1 -> doutsl1_1/2(data_in)
  Convert6/1 -> doutsl1_2/2(data_in)
  Convert7/1 -> doutsl1_3/2(data_in)
  Delay4/1 -> Delay74/1
  Delay10/1 -> Delay73/1
  Delay12/1 -> Delay71/1
  Delay21/1 -> Delay72/1
  Delay32/1 -> Delay70/1
  Delay37/1 -> Delay69/1
  Delay46/1 -> Delay67/1
  Delay38/1 -> Delay68/1
  Delay51/1 -> Delay77/1
  Delay50/1 -> Delay78/1
  Delay52/1 -> Delay76/1
  Delay54/1 -> Delay75/1
  Delay53/1 -> Delay82/1
  Delay47/1 -> Delay81/1
  Delay48/1 -> Delay79/1
  Delay49/1 -> Delay80/1
  Delay55/1 -> Delay65/1
  Delay56/1 -> Delay66/1
  freeze_cntr3/3 -> Terminator39/1
  [trigger] -> freeze_cntr3/2
  Delay57/1 -> Delay60/1
  Mult/1 -> Slice11/1
  freeze_cntr3/1 -> dout_denom_0/1(addr), dout_denom_1/1(addr), dout_denom_2/1(addr), dout_denom_3/1(addr)
  freeze_cntr3/2 -> dout_denom_0/3(we), dout_denom_1/3(we), dout_denom_2/3(we), dout_denom_3/3(we)
  Mult1/1 -> Slice12/1
  Mult2/1 -> Slice13/1
  Mult3/1 -> Slice14/1
  dout_denom_0/1(data_out) -> Terminator26/1
  dout_denom_2/1(data_out) -> Terminator30/1
  dout_denom_1/1(data_out) -> Terminator10/1
  dout_denom_3/1(data_out) -> Terminator32/1
  pipeline34/1(q) -> Terminator43/1
  pipeline36/1(q) -> Terminator42/1
  pipeline38/1(q) -> Terminator41/1
  pipeline154/1(q) -> Terminator46/1
  pipeline156/1(q) -> Terminator45/1
  pipeline158/1(q) -> Terminator44/1
  freeze_cntr4/3 -> Terminator51/1
  [trigger] -> freeze_cntr4/2
  freeze_cntr4/1 -> dout_num_1/1(addr), dout_num_3/1(addr), dout_num_2/1(addr), dout_num_0/1(addr)
  freeze_cntr4/2 -> dout_num_1/3(we), dout_num_3/3(we), dout_num_2/3(we), dout_num_0/3(we)
  dout_num_0/1(data_out) -> Terminator48/1
  dout_num_2/1(data_out) -> Terminator49/1
  dout_num_1/1(data_out) -> Terminator47/1
  dout_num_3/1(data_out) -> Terminator50/1
  Delay45/1 -> Delay59/1, Delay14/1
  Power/1(Out1) -> Slice15/1
  Slice15/1 -> Divide/1, Delay18/1
  signed_shifter8/1 -> Power/1(In1)
  signed_shifter10/1 -> Power1/1(In1)
  signed_shifter11/1 -> Power1/2(In2)
  signed_shifter14/1 -> Power2/1(In1)
  signed_shifter15/1 -> Power2/2(In2)
  signed_shifter12/1 -> Power3/1(In1)
  signed_shifter13/1 -> Power3/2(In2)
  signed_shifter9/1 -> Power/2(In2)
  Slice11/1 -> Divide/2, Delay61/1
  Slice12/1 -> Divide1/2, Delay62/1
  Slice13/1 -> Divide2/2, Delay63/1
  Slice14/1 -> Divide3/2, Delay64/1
  Power1/1(Out1) -> Slice16/1
  Power2/1(Out1) -> Slice17/1
  Power3/1(Out1) -> Slice18/1
  freeze_cntr5/3 -> Terminator37/1
  freeze_cntr5/1 -> dout_score_1/1(addr), dout_score_3/1(addr), dout_score_2/1(addr), dout_score_0/1(addr)
  freeze_cntr5/2 -> dout_score_1/3(we), dout_score_3/3(we), dout_score_2/3(we), dout_score_0/3(we)
  dout_score_0/1(data_out) -> Terminator34/1
  dout_score_2/1(data_out) -> Terminator35/1
  dout_score_1/1(data_out) -> Terminator33/1
  dout_score_3/1(data_out) -> Terminator36/1
  Divide1/1 -> Convert13/1
  Divide/1 -> Convert12/1
  Divide2/1 -> Convert14/1
  Divide3/1 -> Convert15/1
  [trigger] -> freeze_cntr5/2
  Convert12/1 -> Reinterpret/1
  Reinterpret/1 -> dout_score_0/2(data_in)
  Reinterpret8/1 -> dout_score_1/2(data_in)
  Convert13/1 -> Reinterpret8/1
  Reinterpret9/1 -> dout_score_2/2(data_in)
  Reinterpret10/1 -> dout_score_3/2(data_in)
  Convert14/1 -> Reinterpret9/1
  Convert15/1 -> Reinterpret10/1
  Slice16/1 -> Divide1/1, Delay23/1
  Slice17/1 -> Divide2/1, Delay26/1
  Slice18/1 -> Divide3/1, Delay58/1
  Delay14/1 -> freeze_cntr5/1
  Delay18/1 -> dout_num_0/2(data_in)
  Delay23/1 -> dout_num_1/2(data_in)
  Delay58/1 -> dout_num_3/2(data_in)
  Delay26/1 -> dout_num_2/2(data_in)
  Delay59/1 -> freeze_cntr4/1
  Delay60/1 -> freeze_cntr3/1
  Delay61/1 -> dout_denom_0/2(data_in)
  Delay62/1 -> dout_denom_1/2(data_in)
  Delay63/1 -> dout_denom_2/2(data_in)
  Delay64/1 -> dout_denom_3/2(data_in)
  Delay65/1 -> freeze_cntr1/1
  Delay66/1 -> freeze_cntr2/1
  Delay67/1 -> dout1_0/2(data_in)
  Delay68/1 -> dout1_1/2(data_in)
  Delay69/1 -> dout1_2/2(data_in)
  Delay70/1 -> dout1_3/2(data_in)
  Delay71/1 -> Convert4/1
  Delay72/1 -> Convert5/1
  Delay73/1 -> Convert6/1
  Delay74/1 -> Convert7/1
  Delay75/1 -> dout0_0/2(data_in)
  Delay76/1 -> dout0_1/2(data_in)
  Delay78/1 -> dout0_3/2(data_in)
  Delay77/1 -> dout0_2/2(data_in)
  Delay79/1 -> Convert/1
  Delay80/1 -> Convert1/1
  Delay82/1 -> Convert3/1
  Delay81/1 -> Convert2/1
